simulink model "ROMmodel_C2"  (R2023a) (continued)
== system ROMmodel_C2/Model Outputs/IVD Force Readings == (continued)
  Multiply4/1 -> Demux2/1
  Demux6/2 -> IVD Shear forces/1, MATLAB Function/6(f), IVD1 Shear force/1
  Gain10/1 -> IVD4 MPa/1, Scope12/1
  IVD1 area mm2/1 -> Divide4/2
  IVD5 area mm2/1 -> Divide/2
  Multiply8/1 -> Demux6/1
  Add12/1 -> Multiply5/1
  Add15/1 -> Multiply8/1
  Divide/1 -> Gain9/1
  Gain11/1 -> IVD3 MPa/1, Scope13/1
  Demux4/2 -> IVD Shear forces/3, MATLAB Function/8(h), IVD3 Shear force/1
  Multiply5/1 -> Demux3/1
  Divide2/1 -> Gain11/1
  Divide3/1 -> Gain12/1
  MATLAB Function/1(SumofSquareofIVDForces) -> Minimize SumofSquareofIVDForces/1, Display7/1  "Cost3"
  Demux3/3 -> MATLAB Function/4(d), IVD4 Normal force/1, Divide1/1
  In10/1 -> Add11/2
  Demux6/3 -> MATLAB Function/1(a), IVD1 Normal force/1, Divide4/1
  Demux2/2 -> IVD Shear forces/5, MATLAB Function/10(j), IVD5 Shear force/1
  In5/1 -> Add13/1
  Divide4/1 -> Gain13/1
  Gain9/1 -> IVD5 MPa/1, Scope11/1
  In9/1 -> Add11/1
  Add11/1 -> Multiply4/1
  Gain13/1 -> IVD1 MPa/1, Scope15/1
  In3/1 -> Add14/1
  In4/1 -> Add14/2
  In6/1 -> Add13/2
  In1/1 -> Add15/1
  In8/1 -> Add12/2
  In2/1 -> Add15/2

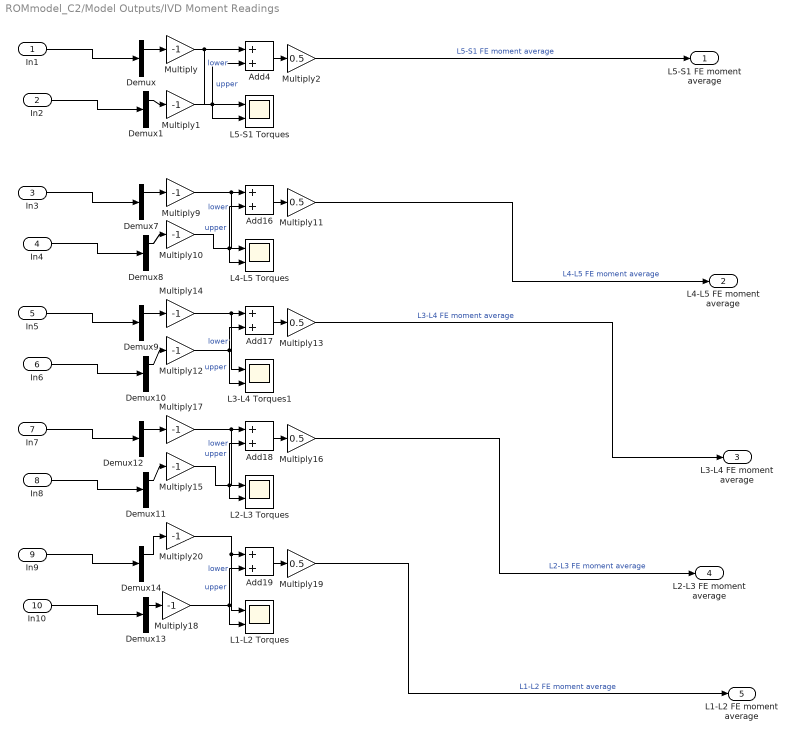
== system ROMmodel_C2/Model Outputs/IVD Moment Readings ==
  In1 : Inport
  In2 : Inport  Port=2
  In3 : Inport  Port=3
  In4 : Inport  Port=4
  In5 : Inport  Port=5
  In6 : Inport  Port=6
  In7 : Inport  Port=7
  In8 : Inport  Port=8
  In9 : Inport  Port=9
  In10 : Inport  Port=10
  Add{4,16..19} (5 alike) : Sum
  Demux{,1,7..14} (10 alike) : Demux  Outputs=3
  L1-L2 Torques : Scope  NumInputPorts=2
  L2-L3 Torques : Scope  NumInputPorts=2
  L3-L4 Torques1 : Scope  NumInputPorts=2
  L4-L5 Torques : Scope  NumInputPorts=2
  L5-S1 Torques : Scope  NumInputPorts=2
  Multiply{,1,9..10,12,14..15,17..18,20} (10 alike) : Gain  Gain=-1
  Multiply{2,11,13,16,19} (5 alike) : Gain  Gain=0.5
  L5-S1 FE moment average : Outport
  L4-L5 FE moment average : Outport  Port=2
  L3-L4 FE moment average : Outport  Port=3
  L2-L3 FE moment average : Outport  Port=4
  L1-L2 FE moment average : Outport  Port=5
  wires:
  Multiply13/1 -> L3-L4 FE moment average/1  "L3-L4 FE moment average"
  Multiply18/1 -> L1-L2 Torques/2, Add19/2  "upper"
  In5/1 -> Demux9/1
  Multiply15/1 -> L2-L3 Torques/2, Add18/2  "upper"
  Add16/1 -> Multiply11/1
  Multiply1/1 -> L5-S1 Torques/2, Add4/2  "upper"
  In4/1 -> Demux8/1
  Multiply20/1 -> Add19/1, L1-L2 Torques/1  "lower"
  Multiply10/1 -> L4-L5 Torques/2, Add16/2  "upper"
  Multiply12/1 -> L3-L4 Torques1/2, Add17/2  "upper"
  Add18/1 -> Multiply16/1
  Multiply2/1 -> L5-S1 FE moment average/1  "L5-S1 FE moment average"
  Add17/1 -> Multiply13/1
  Demux12/1 -> Multiply17/1
  Multiply17/1 -> Add18/1, L2-L3 Torques/1  "lower"
  Demux1/1 -> Multiply1/1
  Demux10/1 -> Multiply12/1
  Demux14/1 -> Multiply20/1
  Multiply16/1 -> L2-L3 FE moment average/1  "L2-L3 FE moment average"
  Demux9/1 -> Multiply14/1
  Add19/1 -> Multiply19/1
  Multiply14/1 -> Add17/1, L3-L4 Torques1/1  "lower"
  Multiply9/1 -> Add16/1, L4-L5 Torques/1  "lower"
  In2/1 -> Demux1/1
  In7/1 -> Demux12/1
  In3/1 -> Demux7/1
  Multiply/1 -> Add4/1, L5-S1 Torques/1  "lower"
  Add4/1 -> Multiply2/1
  In1/1 -> Demux/1
  Demux/1 -> Multiply/1
  Demux11/1 -> Multiply15/1
  Demux8/1 -> Multiply10/1
  Demux13/1 -> Multiply18/1
  In8/1 -> Demux11/1
  Demux7/1 -> Multiply9/1
  Multiply19/1 -> L1-L2 FE moment average/1  "L1-L2 FE moment average"
  Multiply11/1 -> L4-L5 FE moment average/1  "L4-L5 FE moment average"
  In6/1 -> Demux10/1
  In9/1 -> Demux14/1
  In10/1 -> Demux13/1

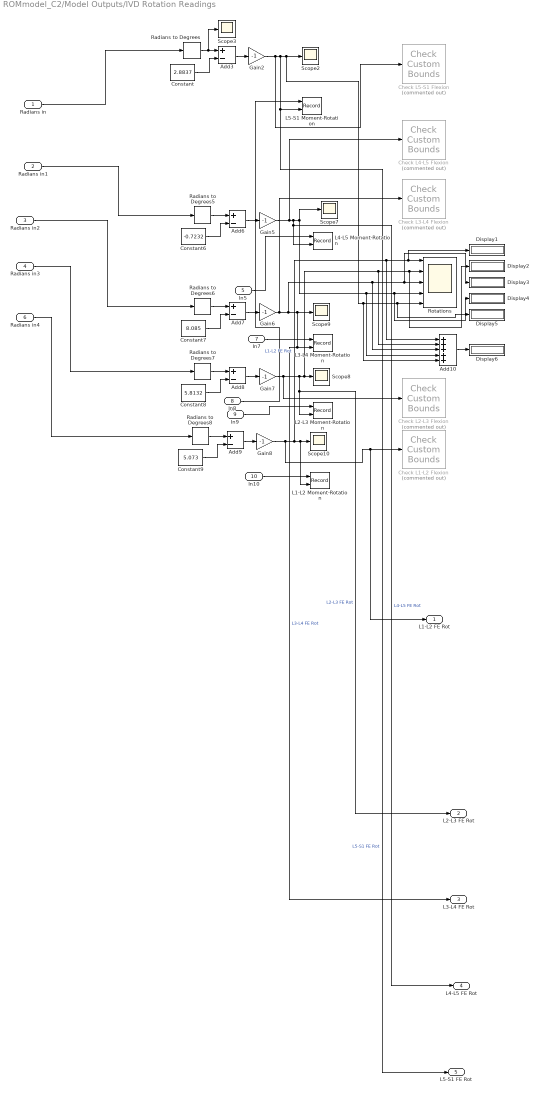
== system ROMmodel_C2/Model Outputs/IVD Rotation Readings ==
  Radians in : Inport
  Radians in1 : Inport  Port=2
  Radians in2 : Inport  Port=3
  Radians in3 : Inport  Port=4
  In5 : Inport  Port=5
  Radians in4 : Inport  Port=6
  In7 : Inport  Port=7
  In8 : Inport  Port=8
  In9 : Inport  Port=9
  In10 : Inport  Port=10
  Add10 : Sum  Inputs=+++++
  Add{3,6..9} (5 alike) : Sum  Inputs=+-
  Check L1-L2 Flexion : checkpacklib/Check Custom Bounds  Commented=on  InstantiateOnLoad=on  enabled=off  stopWhenAssertionFail=off  export=off  ViewDlgPos=[697 199.4 508.8 390.4]  OpenViewOnLoad=off  LaunchViewOnOpen=off  TimeUnits=seconds  EnableUpperBound=on  UpperBoundTimes=[0.54 0.56]  UpperBoundAmplitudes=[10.8 10.8]  EnableLowerBound=on  LowerBoundTimes=[0.54 0.56]  LowerBoundAmplitudes=[6.8 6.8]
      settings: LibrarySourceBlock=sdolib/Signal Constraints/Check Custom Bounds
  Check L2-L3 Flexion : checkpacklib/Check Custom Bounds  Commented=on  InstantiateOnLoad=on  enabled=off  stopWhenAssertionFail=off  export=off  ViewDlgPos=[1119 206 510 390]  OpenViewOnLoad=off  LaunchViewOnOpen=off  TimeUnits=seconds  EnableUpperBound=on  UpperBoundTimes=[0.54 0.56]  UpperBoundAmplitudes=[9.9 9.9]  EnableLowerBound=on  LowerBoundTimes=[0.54 0.56]  LowerBoundAmplitudes=[7.3 7.3]
      settings: LibrarySourceBlock=sdolib/Signal Constraints/Check Custom Bounds
  Check L3-L4 Flexion : checkpacklib/Check Custom Bounds  Commented=on  InstantiateOnLoad=on  enabled=off  stopWhenAssertionFail=off  export=off  ViewDlgPos=[1119 206 510 390]  OpenViewOnLoad=off  LaunchViewOnOpen=off  TimeUnits=seconds  EnableUpperBound=on  UpperBoundTimes=[0.54 0.56]  UpperBoundAmplitudes=[8.8 8.8]  EnableLowerBound=on  LowerBoundTimes=[0.54 0.56]  LowerBoundAmplitudes=[6.4 6.4]
      settings: LibrarySourceBlock=sdolib/Signal Constraints/Check Custom Bounds
  Check L4-L5 Flexion : checkpacklib/Check Custom Bounds  Commented=on  InstantiateOnLoad=on  enabled=off  stopWhenAssertionFail=off  export=off  ViewDlgPos=[1119 206 510 390]  OpenViewOnLoad=off  LaunchViewOnOpen=off  TimeUnits=seconds  EnableUpperBound=on  UpperBoundTimes=[0.54 0.56]  UpperBoundAmplitudes=[7.1 7.1]  EnableLowerBound=on  LowerBoundTimes=[0.54 0.56]  LowerBoundAmplitudes=[4.3 4.3]
      settings: LibrarySourceBlock=sdolib/Signal Constraints/Check Custom Bounds
  Check L5-S1 Flexion : checkpacklib/Check Custom Bounds  Commented=on  InstantiateOnLoad=on  enabled=off  stopWhenAssertionFail=off  export=off  ViewDlgPos=[2337 185 510 390]  OpenViewOnLoad=off  LaunchViewOnOpen=off  TimeUnits=seconds  EnableUpperBound=on  UpperBoundTimes=[0.54 0.56]  UpperBoundAmplitudes=[4.7 4.7]  EnableLowerBound=on  LowerBoundTimes=[0.54 0.56]  LowerBoundAmplitudes=[2.7 2.7]
      settings: LibrarySourceBlock=sdolib/Signal Constraints/Check Custom Bounds
  Constant : Constant  Value=2.8837
  Constant6 : Constant  Value=-0.7232
  Constant7 : Constant  Value=8.085
  Constant8 : Constant  Value=5.8132
  Constant9 : Constant  Value=5.073
  Display{1..6} (6 alike) : Display
  Gain{2,5..8} (5 alike) : Gain  Gain=-1
  L1-L2 Moment-Rotation : Record  FileSettingsInternal="{\"entries\":[{\"content\":{\"excelSettings\":{\"content\":{},\"type\":\"Streamout.ExcelSettings\"}},\"type\":\"Streamout.FileSettingStorage\",\"uuid\":\"05ccfa0e-6c48-43ba-9f44-3c489776dfd3\"}],\"packageUris\":[\"http://schema.mathworks.com/mf0/streamout_blocks/R2020a\"],\"version\":\"1.0\"}"  Layout=[1 1]  SignalsInternal="{\"entries\":[{\"content\":{\"signals\":[{\"content\":{\"blockPath\":[\"ROMmodel_C2/Model Outputs/IVD Rotation Readings/L1-L2 Moment-Rotation\"],\"dimensions\":[1,1],\"domain\":\"ROMmodel_C2/Model Outputs/IVD Rotation Readings/L1-L2 Moment-Rotation\",\"lineColor\":\"#0072bd\",\"port\":1,\"signalID\":356,\"signalName\":\"In10\"},\"type\":\"RecordBlkView.Signal\",\"uuid\":\"97fbaf16-d247-415e-b81e-0de33e5663c8\"},{\"content\":{\"blockPath\":[\"ROMmodel_C2/Model Outputs/IVD Rotation Readings/L1-L2 Moment-Rotation\"],\"dimensions\":[1,1],\"domain\":\"ROMmodel_C2/Model Outputs/IVD Rotation Readings/L1-L2 Moment-Rotation\",\"lineColor\":\"#d95319\",\"port\":2,\"signalID\":359,\"signalName\":\"L1-L2 FE Rot\"},\"type\":\"RecordBlkView.Signal\",\"uuid\":\"33510840-21d6-4966-a015-6eb1dee20ebb\"}]},\"type\":\"RecordBlkView.InputSignals\",\"uuid\":\"339ba31f-4a57-48e4-a65d-239c33e89adc\"}],\"packageUris\":[\"http://schema.mathworks.com/mf0/streamout_blocks/R2020a\"],\"version\":\"1.0\"}"  VisualizationBindings="{\"xyplotplugin\":{\"info\":[{\"series\":[],\"subplotID\":1}]}}"  CompatibilityTag=XY
  L2-L3 Moment-Rotation : Record  FileSettingsInternal="{\"entries\":[{\"content\":{\"excelSettings\":{\"content\":{},\"type\":\"Streamout.ExcelSettings\"}},\"type\":\"Streamout.FileSettingStorage\",\"uuid\":\"74383c56-b9a7-42b6-b201-624be3727690\"}],\"packageUris\":[\"http://schema.mathworks.com/mf0/streamout_blocks/R2020a\"],\"version\":\"1.0\"}"  Layout=[1 1]  SignalsInternal="{\"entries\":[{\"content\":{\"signals\":[{\"content\":{\"blockPath\":[\"ROMmodel_C2/Model Outputs/IVD Rotation Readings/L2-L3 Moment-Rotation\"],\"dimensions\":[1,1],\"domain\":\"ROMmodel_C2/Model Outputs/IVD Rotation Readings/L2-L3 Moment-Rotation\",\"lineColor\":\"#edb120\",\"port\":1,\"signalID\":372,\"signalName\":\"In9\"},\"type\":\"RecordBlkView.Signal\",\"uuid\":\"7261e965-100a-4d92-ae2b-a377c79e83c2\"},{\"content\":{\"blockPath\":[\"ROMmodel_C2/Model Outputs/IVD Rotation Readings/L2-L3 Moment-Rotation\"],\"dimensions\":[1,1],\"domain\":\"ROMmodel_C2/Model Outputs/IVD Rotation Readings/L2-L3 Moment-Rotation\",\"lineColor\":\"#7e2f8e\",\"port\":2,\"signalID\":375,\"signalName\":\"L2-L3 FE Rot\"},\"type\":\"RecordBlkView.Signal\",\"uuid\":\"9ac231ed-3fac-4286-8c1f-1e0d848ef26a\"}]},\"type\":\"RecordBlkView.InputSignals\",\"uuid\":\"bab2dd8c-0f29-4d29-b6a3-1ec88c056b82\"}],\"packageUris\":[\"http://schema.mathworks.com/mf0/streamout_blocks/R2020a\"],\"version\":\"1.0\"}"  VisualizationBindings="{\"xyplotplugin\":{\"info\":[{\"series\":[],\"subplotID\":1}]}}"  CompatibilityTag=XY
  L3-L4 Moment-Rotation : Record  FileSettingsInternal="{\"entries\":[{\"content\":{\"excelSettings\":{\"content\":{},\"type\":\"Streamout.ExcelSettings\"}},\"type\":\"Streamout.FileSettingStorage\",\"uuid\":\"acb6af37-7c2e-437f-8eaf-f8d23155445c\"}],\"packageUris\":[\"http://schema.mathworks.com/mf0/streamout_blocks/R2020a\"],\"version\":\"1.0\"}"  Layout=[1 1]  SignalsInternal="{\"entries\":[{\"content\":{\"signals\":[{\"content\":{\"blockPath\":[\"ROMmodel_C2/Model Outputs/IVD Rotation Readings/L3-L4 Moment-Rotation\"],\"dimensions\":[1,1],\"domain\":\"ROMmodel_C2/Model Outputs/IVD Rotation Readings/L3-L4 Moment-Rotation\",\"lineColor\":\"#77ac30\",\"port\":1,\"signalID\":340,\"signalName\":\"In7\"},\"type\":\"RecordBlkView.Signal\",\"uuid\":\"9f5ce4e6-308b-44fc-9711-8acb4c2d741a\"},{\"content\":{\"blockPath\":[\"ROMmodel_C2/Model Outputs/IVD Rotation Readings/L3-L4 Moment-Rotation\"],\"dimensions\":[1,1],\"domain\":\"ROMmodel_C2/Model Outputs/IVD Rotation Readings/L3-L4 Moment-Rotation\",\"lineColor\":\"#4dbeee\",\"port\":2,\"signalID\":343,\"signalName\":\"L3-L4 FE Rot\"},\"type\":\"RecordBlkView.Signal\",\"uuid\":\"e87dd27f-4dda-4749-aeaa-68231454f0e9\"}]},\"type\":\"RecordBlkView.InputSignals\",\"uuid\":\"79338dff-f6e7-4db4-9346-731cf14e5a50\"}],\"packageUris\":[\"http://schema.mathworks.com/mf0/streamout_blocks/R2020a\"],\"version\":\"1.0\"}"  VisualizationBindings="{\"xyplotplugin\":{\"info\":[{\"series\":[],\"subplotID\":1}]}}"  CompatibilityTag=XY
  L4-L5 Moment-Rotation : Record  FileSettingsInternal="{\"entries\":[{\"content\":{\"excelSettings\":{\"content\":{},\"type\":\"Streamout.ExcelSettings\"}},\"type\":\"Streamout.FileSettingStorage\",\"uuid\":\"419692e5-aaa1-4fac-9047-d3cb98bacc03\"}],\"packageUris\":[\"http://schema.mathworks.com/mf0/streamout_blocks/R2020a\"],\"version\":\"1.0\"}"  Layout=[1 1]  SignalsInternal="{\"entries\":[{\"content\":{\"signals\":[{\"content\":{\"blockPath\":[\"ROMmodel_C2/Model Outputs/IVD Rotation Readings/L4-L5 Moment-Rotation\"],\"dimensions\":[1,1],\"domain\":\"ROMmodel_C2/Model Outputs/IVD Rotation Readings/L4-L5 Moment-Rotation\",\"lineColor\":\"#a2142f\",\"port\":1,\"signalID\":348,\"signalName\":\"In5\"},\"type\":\"RecordBlkView.Signal\",\"uuid\":\"6a612c22-d2e5-43cb-8942-07a0fe5f9162\"},{\"content\":{\"blockPath\":[\"ROMmodel_C2/Model Outputs/IVD Rotation Readings/L4-L5 Moment-Rotation\"],\"dimensions\":[1,1],\"domain\":\"ROMmodel_C2/Model Outputs/IVD Rotation Readings/L4-L5 Moment-Rotation\",\"lineColor\":\"#139fff\",\"port\":2,\"signalID\":351,\"signalName\":\"L4-L5 FE Rot\"},\"type\":\"RecordBlkView.Signal\",\"uuid\":\"31af6b7d-ee4f-4804-a99f-b76e6d559725\"}]},\"type\":\"RecordBlkView.InputSignals\",\"uuid\":\"0bd79b0b-7966-4bc8-b677-8bdfa02a40d3\"}],\"packageUris\":[\"http://schema.mathworks.com/mf0/streamout_blocks/R2020a\"],\"version\":\"1.0\"}"  VisualizationBindings="{\"xyplotplugin\":{\"info\":[{\"series\":[],\"subplotID\":1}]}}"  CompatibilityTag=XY
  L5-S1 Moment-Rotation : Record  FileSettingsInternal="{\"entries\":[{\"content\":{\"excelSettings\":{\"content\":{},\"type\":\"Streamout.ExcelSettings\"}},\"type\":\"Streamout.FileSettingStorage\",\"uuid\":\"b88c74e2-3abb-43ce-bf96-ddd9a6ee6ba9\"}],\"packageUris\":[\"http://schema.mathworks.com/mf0/streamout_blocks/R2020a\"],\"version\":\"1.0\"}"  Layout=[1 1]  SignalsInternal="{\"entries\":[{\"content\":{\"signals\":[{\"content\":{\"blockPath\":[\"ROMmodel_C2/Model Outputs/IVD Rotation Readings/L5-S1 Moment-Rotation\"],\"dimensions\":[1,1],\"domain\":\"ROMmodel_C2/Model Outputs/IVD Rotation Readings/L5-S1 Moment-Rotation\",\"lineColor\":\"#ff6929\",\"port\":1,\"signalID\":364,\"signalName\":\"In8\"},\"type\":\"RecordBlkView.Signal\",\"uuid\":\"2a46c869-168b-4667-a98c-0535738c23b7\"},{\"content\":{\"blockPath\":[\"ROMmodel_C2/Model Outputs/IVD Rotation Readings/L5-S1 Moment-Rotation\"],\"dimensions\":[1,1],\"domain\":\"ROMmodel_C2/Model Outputs/IVD Rotation Readings/L5-S1 Moment-Rotation\",\"lineColor\":\"#b746ff\",\"port\":2,\"signalID\":367,\"signalName\":\"L5-S1 FE Rot\"},\"type\":\"RecordBlkView.Signal\",\"uuid\":\"66f72287-fb77-4b76-85f5-0566e833923a\"}]},\"type\":\"RecordBlkView.InputSignals\",\"uuid\":\"f1b91229-6c06-4eee-a2fd-c10c0c82324b\"}],\"packageUris\":[\"http://schema.mathworks.com/mf0/streamout_blocks/R2020a\"],\"version\":\"1.0\"}"  VisualizationBindings="{\"xyplotplugin\":{\"info\":[{\"series\":[],\"subplotID\":1}]}}"  CompatibilityTag=XY
  Radians to Degrees{,5..8} (5 alike) : simulink_extras/Transformations/Radians to Degrees
  Rotations : Scope  NumInputPorts=5
  Scope{2..3,7..10} (6 alike) : Scope
  L1-L2 FE Rot : Outport
  L2-L3 FE Rot : Outport  Port=2
  L3-L4 FE Rot : Outport  Port=3
  L4-L5 FE Rot : Outport  Port=4
  L5-S1 FE Rot : Outport  Port=5
  wires:
  Add10/1 -> Display6/1
  Gain8/1 -> L1-L2 FE Rot/1, Check L1-L2 Flexion/1, Add10/1, Display1/1, Rotations/1, L1-L2 Moment-Rotation/2, Scope10/1  "L1-L2 FE Rot"
  Add7/1 -> Gain6/1
  Radians to Degrees7/1 -> Add8/1
  Add3/1 -> Gain2/1
  Add6/1 -> Gain5/1
  Constant/1 -> Add3/2
  Gain5/1 -> Check L4-L5 Flexion/1, L4-L5 Moment-Rotation/2, L4-L5 FE Rot/1, Add10/4, Display4/1, Rotations/4, Scope7/1  "L4-L5 FE Rot"
  Gain2/1 -> Check L5-S1 Flexion/1, L5-S1 FE Rot/1, L5-S1 Moment-Rotation/2, Add10/5, Display5/1, Rotations/5, Scope2/1  "L5-S1 FE Rot"
  Radians in1/1 -> Radians to Degrees5/1
  Gain6/1 -> Check L3-L4 Flexion/1, Add10/3, Display3/1, Rotations/3, L3-L4 FE Rot/1, L3-L4 Moment-Rotation/2, Scope9/1  "L3-L4 FE Rot"
  Gain7/1 -> Check L2-L3 Flexion/1, L2-L3 FE Rot/1, L2-L3 Moment-Rotation/2, Add10/2, Display2/1, Rotations/2, Scope8/1  "L2-L3 FE Rot"
  Constant6/1 -> Add6/2
  In9/1 -> L2-L3 Moment-Rotation/1
  Radians to Degrees5/1 -> Add6/1
  Constant9/1 -> Add9/2
  Radians to Degrees6/1 -> Add7/1
  Constant8/1 -> Add8/2
  Add8/1 -> Gain7/1
  Radians to Degrees8/1 -> Add9/1
  Radians in3/1 -> Radians to Degrees7/1
  Constant7/1 -> Add7/2
  Add9/1 -> Gain8/1
  Radians to Degrees/1 -> Scope3/1, Add3/1
  Radians in/1 -> Radians to Degrees/1
  Radians in4/1 -> Radians to Degrees8/1
  Radians in2/1 -> Radians to Degrees6/1
  In7/1 -> L3-L4 Moment-Rotation/1
  In8/1 -> L5-S1 Moment-Rotation/1
  In5/1 -> L4-L5 Moment-Rotation/1
  In10/1 -> L1-L2 Moment-Rotation/1

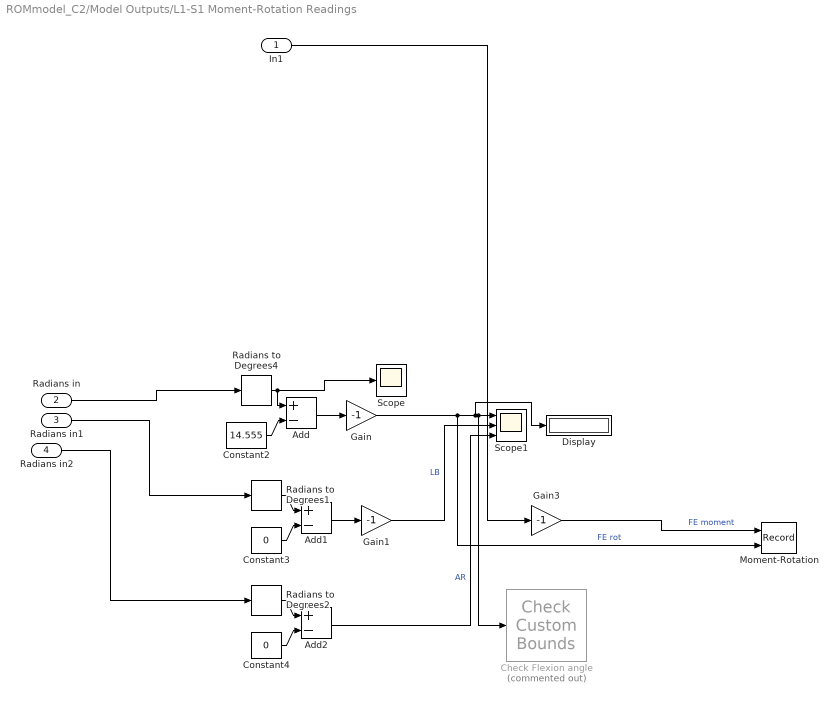
== system ROMmodel_C2/Model Outputs/L1-S1 Moment-Rotation Readings ==
  In1 : Inport
  Radians in : Inport  Port=2
  Radians in1 : Inport  Port=3
  Radians in2 : Inport  Port=4
  Add : Sum  Inputs=+-
  Add1 : Sum  Inputs=+-
  Add2 : Sum  Inputs=+-
  Check Flexion angle : checkpacklib/Check Custom Bounds  Commented=on  InstantiateOnLoad=on  enabled=off  stopWhenAssertionFail=off  export=off  ViewDlgPos=[696 366 509 389]  OpenViewOnLoad=off  LaunchViewOnOpen=on  TimeUnits=seconds  EnableUpperBound=on  UpperBoundTimes=[0 1]  UpperBoundAmplitudes=[5 5];  EnableLowerBound=on  LowerBoundTimes=[0 1]  LowerBoundAmplitudes=[-5 -5];
      settings: LibrarySourceBlock=sdolib/Signal Constraints/Check Custom Bounds
  Constant2 : Constant  Value=14.555
  Constant3 : Constant  Value=0
  Constant4 : Constant  Value=0
  Display : Display
  Gain : Gain  Gain=-1
  Gain1 : Gain  Gain=-1
  Gain3 : Gain  Gain=-1
  Moment-Rotation : Record  FileSettingsInternal="{\"entries\":[{\"content\":{\"excelSettings\":{\"content\":{},\"type\":\"Streamout.ExcelSettings\"}},\"type\":\"Streamout.FileSettingStorage\",\"uuid\":\"d16e91a0-5152-4a81-bc68-d9feb48fab0b\"}],\"packageUris\":[\"http://schema.mathworks.com/mf0/streamout_blocks/R2020a\"],\"version\":\"1.0\"}"  Layout=[1 1]  SignalsInternal="{\"entries\":[{\"content\":{\"signals\":[{\"content\":{\"blockPath\":[\"ROMmodel_C2/Model Outputs/L1-S1 Moment-Rotation Readings/XY Graph1\"],\"dimensions\":[1],\"domain\":\"ROMmodel_C2/Model Outputs/L1-S1 Moment-Rotation Readings/XY Graph1\",\"lineColor\":\"#64d413\",\"plots\":[1],\"port\":1,\"signalID\":332,\"signalName\":\"FE moment\"},\"type\":\"RecordBlkView.Signal\",\"uuid\":\"dff20435-36ca-4609-9b07-417dca0d5710\"},{\"content\":{\"blockPath\":[\"ROMmodel_C2/Model Outputs/L1-S1 Moment-Rotation Readings/XY Graph1\"],\"dimensions\":[1,1],\"domain\":\"ROMmodel_C2/Model Outputs/L1-S1 Moment-Rotation Readings/XY Graph1\",\"lineColor\":\"#ff13a6\",\"plots\":[1],\"port\":2,\"signalID\":335,\"signalName\":\"FE rot\"},\"type\":\"RecordBlkView.Signal\",\"uuid\":\"26b92028-303d-4104-9c34-2f1c50173239\"}]},\"type\":\"RecordBlkView.InputSignals\",\"uuid\":\"05a06ae0-e37b-46ca-a362-dd3e7eac16ad\"}],\"packageUris\":[\"http://schema.mathworks.com/mf0/streamout_blocks/R2020a\"],\"version\":\"1.0\"}"  VisualizationBindings="{\"xyplotplugin\":{\"info\":[{\"series\":[{\"bindingParametersList\":[{\"parameter\":\"X-Axis\",\"signalID\":332,\"signalName\":\"FE moment\"},{\"parameter\":\"Y-Axis\",\"signalID\":335,\"signalName\":\"FE rot\"}],\"seriesID\":13671}],\"subplotID\":1}]}}"  CompatibilityTag=XY
  Radians to Degrees1 : simulink_extras/Transformations/Radians to Degrees
  Radians to Degrees2 : simulink_extras/Transformations/Radians to Degrees
  Radians to Degrees4 : simulink_extras/Transformations/Radians to Degrees
  Scope : Scope
  Scope1 : Scope  NumInputPorts=3
  wires:
  Add/1 -> Gain/1
  Radians to Degrees2/1 -> Add2/1
  Gain/1 -> Check Flexion angle/1, Scope1/1, Display/1, Moment-Rotation/2  "FE rot"
  Gain1/1 -> Scope1/2  "LB"
  Radians to Degrees4/1 -> Add/1, Scope/1
  Constant4/1 -> Add2/2
  Add1/1 -> Gain1/1
  Radians to Degrees1/1 -> Add1/1
  Constant3/1 -> Add1/2
  Gain3/1 -> Moment-Rotation/1  "FE moment"
  Constant2/1 -> Add/2
  Add2/1 -> Scope1/3  "AR"
  Radians in/1 -> Radians to Degrees4/1
  Radians in2/1 -> Radians to Degrees2/1
  In1/1 -> Gain3/1
  Radians in1/1 -> Radians to Degrees1/1

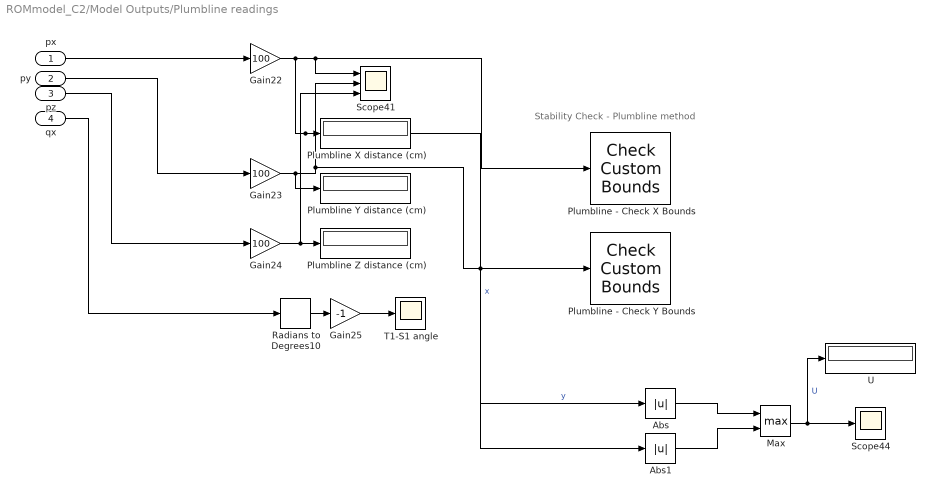
== system ROMmodel_C2/Model Outputs/Plumbline readings ==
  note: Stability Check - Plumbline method
  px : Inport
  py : Inport  Port=2
  pz : Inport  Port=3
  qx : Inport  Port=4
  Abs : Abs  SaturateOnIntegerOverflow=off
  Abs1 : Abs  SaturateOnIntegerOverflow=off
  Gain22 : Gain  Gain=100
  Gain23 : Gain  Gain=100
  Gain24 : Gain  Gain=100
  Gain25 : Gain  Gain=-1
  Max : MinMax  Function=max  Inputs=2
  Plumbline - Check X Bounds : checkpacklib/Check Custom Bounds  InstantiateOnLoad=on  enabled=off  stopWhenAssertionFail=off  export=off  ViewDlgPos=[983.4 172.2 510.4 379.2]  OpenViewOnLoad=off  LaunchViewOnOpen=off  TimeUnits=seconds  EnableUpperBound=on  UpperBoundTimes=[0 1]  UpperBoundAmplitudes=[2 2];  EnableLowerBound=on  LowerBoundTimes=[0 1]  LowerBoundAmplitudes=[-2 -2]
      settings: LibrarySourceBlock=sdolib/Signal Constraints/Check Custom Bounds
  Plumbline - Check Y Bounds : checkpacklib/Check Custom Bounds  InstantiateOnLoad=on  enabled=off  stopWhenAssertionFail=off  export=off  ViewDlgPos=[509 222 510 390]  OpenViewOnLoad=off  LaunchViewOnOpen=off  TimeUnits=seconds  EnableUpperBound=on  UpperBoundTimes=[0 1]  UpperBoundAmplitudes=[2 2];  EnableLowerBound=on  LowerBoundTimes=[0 1]  LowerBoundAmplitudes=[-2 -2]
      settings: LibrarySourceBlock=sdolib/Signal Constraints/Check Custom Bounds
  Plumbline X distance (cm) : Display
  Plumbline Y distance (cm) : Display
  Plumbline Z distance (cm) : Display
  Radians to Degrees10 : simulink_extras/Transformations/Radians to Degrees
  Scope41 : Scope  NumInputPorts=3
  Scope44 : Scope
  T1-S1 angle : Scope
  U : Display
  wires:
  Gain22/1 -> Plumbline - Check X Bounds/1, Scope41/1, Abs1/1, Plumbline X distance (cm)/1  "x"
  Gain23/1 -> Scope41/2, Abs/1, Plumbline - Check Y Bounds/1, Plumbline Y distance (cm)/1  "y"
  Abs/1 -> Max/1
  Gain25/1 -> T1-S1 angle/1
  pz/1 -> Gain24/1
  qx/1 -> Radians to Degrees10/1
  px/1 -> Gain22/1
  Gain24/1 -> Plumbline Z distance (cm)/1, Scope41/3
  Max/1 -> U/1, Scope44/1  "U"
  Abs1/1 -> Max/2
  Radians to Degrees10/1 -> Gain25/1
  py/1 -> Gain23/1

== matlab function ROMmodel_C2/Model Outputs/Cost readings/MATLAB Function ==
  data: Psoas input; SumofCubeofForces output; MF input; LongThor input; EO input; IO input; RA input; SumofMuscleStress output
function [SumofCubeofForces, SumofMuscleStress] = fcn(Psoas,MF,LongThor,EO,IO,RA)

SumofCubeofForces = (1e-6) * (Psoas^3 + MF^3 + LongThor^3 + EO^3 + IO^3 + RA^3) ;



% PCSA from Comparison of muscle forces and joint load from an optimization and EMG assisted lumbar spine model: Towards development of a hybrid approach
% Cholewicki 
% https://doi.org/10.1016/0021-9290(94)00065-C

PCSA_Psoas = 440; % mm^2
PCSA_MF = 1000 ;
PCSA_LongThor = 800 ;
PCSA_EO = 950 ;
PCSA_IO = 850 ;
PCSA_RA = 1000 ;

SumofMuscleStress = (Psoas/PCSA_Psoas) + (MF/PCSA_MF) + (LongThor/PCSA_LongThor) + (EO/PCSA_EO) + (IO/PCSA_IO) + (RA/PCSA_RA) ;  % MPa

== matlab function ROMmodel_C2/Model Outputs/IVD Force Readings/MATLAB Function ==
  data: a input; SumofSquareofIVDForces output; b input; c input; d input; e input; f input; g input; h input; i input; j input
function SumofSquareofIVDForces = fcn(a,b,c,d,e,f,g,h,i,j)

SumofSquareofIVDForces = (1e-5) *(a^2 + b^2 + c^2 + d^2 + e^2 + f^2 + g^2 + h^2 + i^2 + j^2) ; % x 10e-5 N^2
